simulink library "inp"  (Simulink 8.2)
settings: Solver=ode45  SolverMode=SingleTasking  StartTime=0.0  StopTime=10.0  RelTol=1e-3
undefined symbols (not defined in the file or in its folder): C_TICK_TIME_S, ENUM_FAULTSTATE_FAIL, ENUM_FAULTSTATE_INDETERMINATE, ENUM_FAULTSTATE_PASS, ENUM_KEYSWITCH_ON, FALSE, KINP_ABS1CANCommuOk_flg_ovrdflg, KINP_ABS1CANCommuOk_flg_ovrdval, KINP_ABS1CANPeriodTime_s, KINP_ABS1CANRolCntOk_flg_ovrdflg, KINP_ABS1CANRolCntOk_flg_ovrdval, KINP_ABS2CANChkSumOk_flg_ovrdflg, KINP_ABS2CANChkSumOk_flg_ovrdval, KINP_ABS2CANCommuOk_flg_ovrdflg, KINP_ABS2CANCommuOk_flg_ovrdval, KINP_ABS2CANPeriodTime_s, KINP_ABS2CANRolCntOk_flg_ovrdflg, KINP_ABS2CANRolCntOk_flg_ovrdval, KINP_ABS3CANCommuOk_flg_ovrdflg, KINP_ABS3CANCommuOk_flg_ovrdval, KINP_ABS4CANCommuOk_flg_ovrdflg, KINP_ABS4CANCommuOk_flg_ovrdval, KINP_ABSActive_flg_defval, KINP_ABSActive_flg_invalid, KINP_ABSActive_flg_ovrdflg, KINP_ABSActive_flg_ovrdval, KINP_ABSActive_flg_setvalid, KINP_ABSActive_flg_srvcflg, KINP_ABSActive_flg_srvcval, KINP_ABSCANAlive_flg_ovrdflg, KINP_ABSCANAlive_flg_ovrdval, KINP_ABSCANCommuOk_flg_ovrdflg, KINP_ABSCANCommuOk_flg_ovrdval, KINP_ABSCANValidOk_flg_ovrdflg, KINP_ABSCANValidOk_flg_ovrdval, KINP_ABSFault_flg_defval, KINP_ABSFault_flg_invalid, KINP_ABSFault_flg_ovrdflg, KINP_ABSFault_flg_ovrdval, KINP_ABSFault_flg_setvalid, KINP_ABSFault_flg_srvcflg, KINP_ABSFault_flg_srvcval, KINP_AC1CANCommuOk_flg_ovrdflg, KINP_AC1CANCommuOk_flg_ovrdval, KINP_ACCANAlive_flg_ovrdflg, KINP_ACCANAlive_flg_ovrdval, KINP_ACCANCommuOk_flg_ovrdflg, KINP_ACCANCommuOk_flg_ovrdval, KINP_ACCANValidOk_flg_ovrdflg, KINP_ACCANValidOk_flg_ovrdval, KINP_ACCCancelH_flg_defval, KINP_ACCCancelH_flg_invalid, KINP_ACCCancelH_flg_ovrdflg, KINP_ACCCancelH_flg_ovrdval, KINP_ACCCancelH_flg_setvalid, KINP_ACCCancelH_flg_srvcflg, KINP_ACCCancelH_flg_srvcval, KINP_AccelPedalPosn_pct_defval, KINP_AccelPedalPosn_pct_gain, KINP_AccelPedalPosn_pct_invalid ... (+1866)
libraries: inp x192, hcu_lib x34, simulink x33
(part 14 of 21)

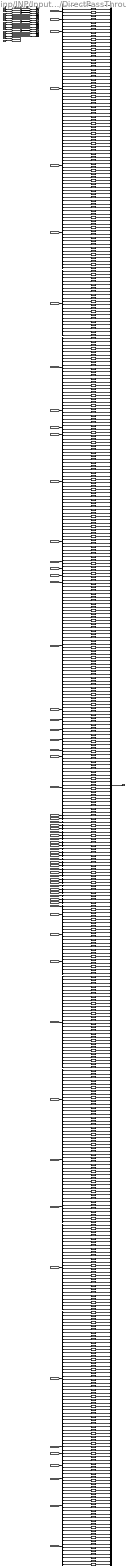
== system inp/INP/InputSignals/DirectPassThrough ==
  ECM_signal_bus : Inport
  TCM_signal_bus : Inport  Port=2
  BCM_signal_bus : Inport  Port=3
  ABS_signal_bus : Inport  Port=4
  EPS_signal_bus : Inport  Port=5
  METER_signal_bus : Inport  Port=6
  AC_signal_bus : Inport  Port=7
  EPB_signal_bus : Inport  Port=8
  CSW_signal_bus : Inport  Port=9
  VCU_signal_bus : Inport  Port=10
  HMI_signal_bus : Inport  Port=11
  EPM_signal_bus : Inport  Port=12
  HWIO_signal_bus : Inport  Port=13
  LLSW_signal_bus : Inport  Port=14
  INP_calc_bus : Inport  Port=15
  INP_diag_bus : Inport  Port=16
  Bus : BusCreator  Inputs=465  OutDataTypeStr=Bus: BINP_outputs
  Bus Selector1 : BusSelector  OutputSignals=VINP_LaneC0LKalF_m_InProc,VINP_LaneC1LKalF_rad_InProc,VINP_LaneC2LKalF_rat_InProc,VINP_LaneC3LKalF_rat_InProc
  Bus Selector10 : BusSelector  OutputSignals=VINP_AckCurBrakDecReq_mps2_InHAL,VINP_AckCurBrakDecReqValid_flg_InHAL,VINP_AckCurBrakDecReqApplable_flg_InHAL,VINP_AckCurBrakDecReqApplableValid_flg_InHAL,VINP_AckCurPTTqReq_Nm_InHAL,VINP_AckCurPTTqReqValid_flg_InHAL,VINP_AckCurPTTqReqApplable_flg_InHAL,VINP_AckCurPTTqReqApplableValid_flg_InHAL,VINP_AckCurVehDec_mps2_InHAL,VINP_AckCurVehDecValid_flg_InHAL,VINP_DeceContManag_flg_InHAL,VINP_DeceContManagValid_flg_InHAL,VINP_DriverBrakPedalOn_flg_InHAL,VINP_DriverBrakPedalOnValid_flg_InHAL,VINP_ECMExtnTqAvailable_flg_InHAL,VINP_ECMExtnTqAvailableValid_flg_InHAL,VINP_ECMTqSource_enum_InHAL,VINP_ECMTqSourceValid_flg_InHAL,VINP_ESCBrakeStatus_enum_InHAL,VINP_ESCBrakeStatusValid_flg_InHAL,VINP_ESCExtnBrkAvailable_flg_InHAL,VINP_ESCExtnBrkAvailableValid_flg_InHAL,VINP_ESCBattVolt_V_InHAL,VINP_ESCBattVoltValid_flg_InHAL,VINP_HWAccelPedalPosn_pct_InHAL,VINP_HWAccelPedalPosnValid_flg_InHAL,VINP_CurDriverTqReq_Nm_InHAL,VINP_CurDriverTqReqValid_flg_InHAL,VINP_TCMGearPosnReqAllow_enum_InHAL,VINP_TCMGearPosnReqAllowValid_flg_InHAL,VINP_MSAStatus_enum_InHAL,VINP_MSAStatusValid_flg_InHAL,VINP_HybridLimpHome_flg_InHAL,VINP_HybridLimpHomeValid_flg_InHAL,VINP_HCUMILReq_flg_InHAL,VINP_HCUMILReqValid_flg_InHAL
  Bus Selector11 : BusSelector  OutputSignals=VINP_ACCTimeGapIncVCU_flg_InHAL,VINP_ACCTimeGapIncVCUValid_flg_InHAL,VINP_ACCTimeGapDecVCU_flg_InHAL,VINP_ACCTimeGapDecVCUValid_flg_InHAL
  Bus Selector12 : BusSelector  OutputSignals=VINP_AEBEnableH_flg_InHAL,VINP_AEBEnableHValid_flg_InHAL,VINP_FCWEnableH_flg_InHAL,VINP_FCWEnableHValid_flg_InHAL,VINP_CSCEnableH_flg_InHAL,VINP_CSCEnableHValid_flg_InHAL,VINP_LKAEnableH_flg_InHAL,VINP_LKAEnableHValid_flg_InHAL,VINP_MAPAEnableH_flg_InHAL,VINP_MAPAEnableHValid_flg_InHAL,VINP_LCSEnableH_flg_InHAL,VINP_LCSEnableHValid_flg_InHAL,VINP_FCWWarningModeH_enum_InHAL,VINP_FCWWarningModeHValid_flg_InHAL,VINP_DefMaxACCSetSpeed_kph_InHAL,VINP_DefMaxACCSetSpeedValid_flg_InHAL
  Bus Selector13 : BusSelector  OutputSignals=VINP_LFCPilotOnHValid_flg_InHAL
  Bus Selector14 : BusSelector  OutputSignals=VINP_LFCPilotOnH_flg_InProc
  Bus Selector15 : BusSelector  OutputSignals=VINP_LFCPilotCancelHValid_flg_InHAL
  Bus Selector16 : BusSelector  OutputSignals=VINP_LFCPilotCancelH_flg_InProc
  Bus Selector17 : BusSelector  OutputSignals=VINP_ACCOnHValid_flg_InHAL
  Bus Selector18 : BusSelector  OutputSignals=VINP_ACCOnH_flg_InProc
  Bus Selector19 : BusSelector  OutputSignals=VINP_ACCCancelHValid_flg_InHAL
  Bus Selector197 : BusSelector  OutputSignals=VINP_IgnitionVolt_V_InHAL,VINP_IgnitionVoltValid_flg_InHAL,VINP_BatteryVolt_V_InHAL,VINP_BatteryVoltValid_flg_InHAL
  Bus Selector2 : BusSelector  OutputSignals=VINP_LaneC0RKalF_m_InProc,VINP_LaneC1RKalF_rad_InProc,VINP_LaneC2RKalF_rat_InProc,VINP_LaneC3RKalF_rat_InProc
  Bus Selector20 : BusSelector  OutputSignals=VINP_ACCCancelH_flg_InProc
  Bus Selector202 : BusSelector  OutputSignals=VINP_IgnitionActive_flg_InProc
  Bus Selector203 : BusSelector  OutputSignals=VHAL_ADCFault_enum,VHAL_EEPROMFault_enum,VHAL_RAMFault_enum,VHAL_ROMFault_enum,VHAL_VREF1Fault_enum,VHAL_VREF2Fault_enum
  Bus Selector204 : BusSelector  OutputSignals=VINP_AccelPedalPosn_pct_InHAL,VINP_AccelPedalPosnValid_flg_InHAL,VINP_EffAccelPedalPosn_pct_InHAL,VINP_EffAccelPedalPosnValid_flg_InHAL,VINP_EngSpd_erpm_InHAL,VINP_EngSpdValid_flg_InHAL,VINP_EngTqFriction_Nm_InHAL,VINP_EngTqFrictionValid_flg_InHAL,VINP_EngTqIndicated_Nm_InHAL,VINP_EngTqIndicatedValid_flg_InHAL,VINP_EngTqIndicatedCOR_Nm_InHAL,VINP_EngTqIndicatedCORValid_flg_InHAL,VINP_EngTqMax_Nm_InHAL,VINP_EngTqMaxValid_flg_InHAL,VINP_EngTqMin_Nm_InHAL,VINP_EngTqMinValid_flg_InHAL,VINP_EngTqUnCORFault_flg_InHAL,VINP_EngTqUnCORFaultValid_flg_InHAL,VINP_EMSHybridOpState_enum_InHAL,VINP_EMSHybridOpStateValid_flg_InHAL,VINP_EMSOpState_enum_InHAL,VINP_EMSOpStateValid_flg_InHAL,VINP_EngHoodOpen_flg_InHAL,VINP_EngHoodOpenValid_flg_InHAL,VINP_OBDWarnCycAchiv_flg_InHAL,VINP_OBDWarnCycAchivValid_flg_InHAL,VINP_OBDDriveCycAchiv_flg_InHAL,VINP_OBDDriveCycAchivValid_flg_InHAL
  Bus Selector209 : BusSelector  OutputSignals=VINP_ABSActive_flg_InHAL,VINP_ABSActiveValid_flg_InHAL,VINP_ABSFault_flg_InHAL,VINP_ABSFaultValid_flg_InHAL,VINP_EBDFault_flg_InHAL,VINP_EBDFaultValid_flg_InHAL,VINP_ESCActive_flg_InHAL,VINP_ESCActiveValid_flg_InHAL,VINP_ESCFault_flg_InHAL,VINP_ESCFaultValid_flg_InHAL,VINP_TCSActive_flg_InHAL,VINP_TCSActiveValid_flg_InHAL,VINP_TCSEnabled_flg_InHAL,VINP_TCSEnabledValid_flg_InHAL,VINP_TCSFault_flg_InHAL,VINP_TCSFaultValid_flg_InHAL,VINP_HSAActive_flg_InHAL,VINP_HSAActiveValid_flg_InHAL,VINP_HSAFault_flg_InHAL,VINP_HSAFaultValid_flg_InHAL
  Bus Selector21 : BusSelector  OutputSignals=VINP_ACCResSpdIncHValid_flg_InHAL
  Bus Selector22 : BusSelector  OutputSignals=VINP_ACCResSpdIncH_flg_InProc
  Bus Selector220 : BusSelector  OutputSignals=VINP_VehSpd_mps_InProc,VINP_SteerAngle_rad_InProc,VINP_YawRate_radps_InProc
  Bus Selector226 : BusSelector  OutputSignals=VINP_KeySwitchStatus_enum_InHAL,VINP_KeySwitchStatusValid_flg_InHAL,VINP_TurnSignalSwL_flg_InHAL,VINP_TurnSignalSwLValid_flg_InHAL,VINP_TurnSignalSwR_flg_InHAL,VINP_TurnSignalSwRValid_flg_InHAL,VINP_HazardLightSw_flg_InHAL,VINP_HazardLightSwValid_flg_InHAL,VINP_DriverDoorOpen_flg_InHAL,VINP_DriverDoorOpenValid_flg_InHAL,VINP_PassengerDoorOpen_flg_InHAL,VINP_PassengerDoorOpenValid_flg_InHAL,VINP_LRDoorOpen_flg_InHAL,VINP_LRDoorOpenValid_flg_InHAL,VINP_RRDoorOpen_flg_InHAL,VINP_RRDoorOpenValid_flg_InHAL,VINP_TailGateOpen_flg_InHAL,VINP_TailGateOpenValid_flg_InHAL,VINP_AnyDoorOpen_flg_InHAL,VINP_AnyDoorOpenValid_flg_InHAL,VINP_VehCrashed_flg_InHAL,VINP_VehCrashedValid_flg_InHAL
  Bus Selector23 : BusSelector  OutputSignals=VINP_ACCSetSpdDecHValid_flg_InHAL
  Bus Selector230 : BusSelector  OutputSignals=VINP_ShifterPosn_enum_InHAL,VINP_ShifterPosnValid_flg_InHAL,VINP_NowGearPosn_enum_InHAL,VINP_NowGearPosnValid_flg_InHAL,VINP_TMTurbineSpd_rpm_InHAL,VINP_TMTurbineSpdValid_flg_InHAL,VINP_TCMFaultStatus_enum_InHAL,VINP_TCMFaultStatusValid_flg_InHAL,VINP_TqConvClutchSt_enum_InHAL,VINP_TqConvClutchStValid_flg_InHAL,VINP_TCMMILReq_flg_InHAL,VINP_TCMMILReqValid_flg_InHAL,VINP_TCMWinterMode_flg_InHAL,VINP_TCMWinterModeValid_flg_InHAL,VINP_TCMPowerMode_flg_InHAL,VINP_TCMPowerModeValid_flg_InHAL,VINP_DriverMode_enum_InHAL,VINP_DriverModeValid_flg_InHAL
  Bus Selector232 : BusSelector  OutputSignals=VINP_RelSteerAngle_deg_InHAL,VINP_RelSteerAngleValid_flg_InHAL,VINP_SteerTorque_Nm_InHAL,VINP_SteerTorqueValid_flg_InHAL,VINP_EPSElectPWR_A_InHAL,VINP_EPSElectPWRValid_flg_InHAL,VINP_EPSFailed_flg_InHAL,VINP_EPSFailedValid_flg_InHAL,VINP_AckCurStrAngReq_deg_InHAL,VINP_AckCurStrAngReqValid_flg_InHAL,VINP_AckCurStrAngReqApplable_flg_InHAL,VINP_AckCurStrAngReqApplableValid_flg_InHAL,VINP_AckCurStrTqReq_Nm_InHAL,VINP_AckCurStrTqReqValid_flg_InHAL,VINP_AckCurStrTqReqApplable_flg_InHAL,VINP_AckCurStrTqReqApplableValid_flg_InHAL,VINP_EPSBattVolt_V_InHAL,VINP_EPSBattVoltValid_flg_InHAL,VINP_EPASMILReq_flg_InHAL,VINP_EPASMILReqValid_flg_InHAL,VINP_EPSLKAStatus_enum_InHAL,VINP_EPSLKAStatusValid_flg_InHAL,VINP_EPSLFCStatus_enum_InHAL,VINP_EPSLFCStatusValid_flg_InHAL,VINP_EPSADASAbReason_enum_InHAL,VINP_EPSADASAbReasonValid_flg_InHAL
  Bus Selector237 : BusSelector  OutputSignals=VINP_LaneQualityL_enum_InHAL,VINP_LaneQualityLValid_flg_InHAL,VINP_LaneQualityR_enum_InHAL,VINP_LaneQualityRValid_flg_InHAL
  Bus Selector239 : BusSelector  OutputSignals=VINP_LaneC0L_m_InHAL,VINP_LaneC0LValid_flg_InHAL,VINP_LaneC1L_rad_InHAL,VINP_LaneC1LValid_flg_InHAL,VINP_LaneC2L_rat_InHAL,VINP_LaneC2LValid_flg_InHAL,VINP_LaneC3L_rat_InHAL,VINP_LaneC3LValid_flg_InHAL
  Bus Selector24 : BusSelector  OutputSignals=VINP_ACCSetSpdDecH_flg_InProc
  Bus Selector240 : BusSelector  OutputSignals=VINP_LaneC0R_m_InHAL,VINP_LaneC0RValid_flg_InHAL,VINP_LaneC1R_rad_InHAL,VINP_LaneC1RValid_flg_InHAL,VINP_LaneC2R_rat_InHAL,VINP_LaneC2RValid_flg_InHAL,VINP_LaneC3R_rat_InHAL,VINP_LaneC3RValid_flg_InHAL
  Bus Selector241 : BusSelector  OutputSignals=VINP_ABSCANValid_flg_InProc,VINP_BCMCANValid_flg_InProc,VINP_ECMCANValid_flg_InProc,VINP_TCMCANValid_flg_InProc,VINP_EPSCANValid_flg_InProc,VINP_METERCANValid_flg_InProc,VINP_ACCANValid_flg_InProc,VINP_EPBCANValid_flg_InProc,VINP_VCUCANValid_flg_InProc,VINP_HMICANValid_flg_InProc,VINP_CSWCANValid_flg_InProc,VINP_EPMCANValid_flg_InProc
  Bus Selector242 : BusSelector  OutputSignals=VINP_ABSCANAlive_flg_InProc,VINP_BCMCANAlive_flg_InProc,VINP_ECMCANAlive_flg_InProc,VINP_TCMCANAlive_flg_InProc,VINP_EPSCANAlive_flg_InProc,VINP_METERCANAlive_flg_InProc,VINP_ACCANAlive_flg_InProc,VINP_EPBCANAlive_flg_InProc,VINP_VCUCANAlive_flg_InProc,VINP_HMICANAlive_flg_InProc,VINP_CSWCANAlive_flg_InProc,VINP_EPMCANAlive_flg_InProc
  Bus Selector25 : BusSelector  OutputSignals=VINP_ACCSpdIncHValid_flg_InHAL
  Bus Selector26 : BusSelector  OutputSignals=VINP_ACCSpdIncH_flg_InProc
  Bus Selector27 : BusSelector  OutputSignals=VINP_ACCSpdDecHValid_flg_InHAL
  Bus Selector28 : BusSelector  OutputSignals=VINP_ACCSpdDecH_flg_InProc
  Bus Selector29 : BusSelector  OutputSignals=VINP_ACCTimeGapIncHValid_flg_InHAL
  Bus Selector3 : BusSelector  OutputSignals=VINP_LFWheelSpeed_kph_InHAL,VINP_LFWheelSpeedValid_flg_InHAL,VINP_LRWheelSpeed_kph_InHAL,VINP_LRWheelSpeedValid_flg_InHAL,VINP_RFWheelSpeed_kph_InHAL,VINP_RFWheelSpeedValid_flg_InHAL,VINP_RRWheelSpeed_kph_InHAL,VINP_RRWheelSpeedValid_flg_InHAL
  Bus Selector30 : BusSelector  OutputSignals=VINP_ACCTimeGapIncH_flg_InProc
  Bus Selector31 : BusSelector  OutputSignals=VINP_ACCTimeGapDecHValid_flg_InHAL
  Bus Selector32 : BusSelector  OutputSignals=VINP_ACCTimeGapDecH_flg_InProc
  Bus Selector33 : BusSelector  OutputSignals=VINP_IGSAOnHValid_flg_InHAL
  Bus Selector34 : BusSelector  OutputSignals=VINP_IGSAOnH_flg_InProc
  Bus Selector35 : BusSelector  OutputSignals=VINP_IGSAResSpdIncHValid_flg_InHAL
  Bus Selector36 : BusSelector  OutputSignals=VINP_IGSAResSpdIncH_flg_InProc
  Bus Selector37 : BusSelector  OutputSignals=VINP_IGSASetSpdDecHValid_flg_InHAL
  Bus Selector38 : BusSelector  OutputSignals=VINP_IGSASetSpdDecH_flg_InProc
  Bus Selector39 : BusSelector  OutputSignals=VINP_IGSASwitchModeHValid_flg_InHAL
  Bus Selector4 : BusSelector  OutputSignals=VINP_AppTime_ms_InProc
  Bus Selector40 : BusSelector  OutputSignals=VINP_IGSASwitchModeH_flg_InProc
  Bus Selector41 : BusSelector  OutputSignals=VINP_SteerAngle_deg_InHAL,VINP_SteerAngleValid_flg_InHAL
  Bus Selector42 : BusSelector  OutputSignals=VINP_SteerSpeed_degps_InProc,VINP_SteerSpeedValid_flg_InProc
  Bus Selector43 : BusSelector  OutputSignals=VINP_HighBeamSwOn_flg_InHAL,VINP_HighBeamSwOnValid_flg_InHAL
  Bus Selector44 : BusSelector  OutputSignals=VINP_ACCResSpdIncVCU_flg_InHAL,VINP_ACCResSpdIncVCUValid_flg_InHAL,VINP_ACCSetSpdDecVCU_flg_InHAL,VINP_ACCSetSpdDecVCUValid_flg_InHAL
  Bus Selector45 : BusSelector  OutputSignals=VINP_ACCOnVCU_flg_InProc,VINP_ACCOnVCUValid_flg_InProc
  Bus Selector46 : BusSelector  OutputSignals=VINP_ACCDistVCU_flg_InProc,VINP_ACCDistVCUValid_flg_InProc
  Bus Selector47 : BusSelector  OutputSignals=VINP_CamNofObjects_num_InHAL,VINP_CamNofObjectsValid_flg_InHAL,VINP_CamGoDecision_enum_InHAL,VINP_CamGoDecisionValid_flg_InHAL,VINP_CloseCarDetected_flg_InHAL,VINP_CloseCarDetectedValid_flg_InHAL,VINP_RightCarCutIn_flg_InHAL,VINP_RightCarCutInValid_flg_InHAL,VINP_LeftCarCutIn_flg_InHAL,VINP_LeftCarCutInValid_flg_InHAL
  Bus Selector48 : BusSelector  OutputSignals=VINP_CamFSImpairedVD_flg_InHAL,VINP_CamFSImpairedVDValid_flg_InHAL,VINP_CamFSLowSun_flg_InHAL,VINP_CamFSLowSunValid_flg_InHAL,VINP_CamFSBlurImage_flg_InHAL,VINP_CamFSBlurImageValid_flg_InHAL,VINP_CamFSSmearImage_flg_InHAL,VINP_CamFSSmearImageValid_flg_InHAL,VINP_CamFSPartialBlockage_flg_InHAL,VINP_CamFSPartialBlockageValid_flg_InHAL,VINP_CamFSFullBlockage_flg_InHAL,VINP_CamFSFullBlockageValid_flg_InHAL,VINP_CamFSSplashes_flg_InHAL,VINP_CamFSSplashesValid_flg_InHAL,VINP_CamFSOutofCal_flg_InHAL,VINP_CamFSOutofCalValid_flg_InHAL,VINP_CamFSCalMisalign_flg_InHAL,VINP_CamFSCalMisalignValid_flg_InHAL,VINP_CamFSSmearedspots_flg_InHAL,VINP_CamFSSmearedspotsValid_flg_InHAL,VINP_CamFSSpotHalosFrozen_flg_InHAL,VINP_CamFSSpotHalosFrozenValid_flg_InHAL,VINP_CamFSFoggySpots_flg_InHAL,VINP_CamFSFoggySpotsValid_flg_InHAL,VINP_CamFSSunRay_flg_InHAL,VINP_CamFSSunRayValid_flg_InHAL
  Bus Selector49 : BusSelector  OutputSignals=VINP_RearFogSwOn_flg_InProc,VINP_RearFogSwOnValid_flg_InProc
  Bus Selector5 : BusSelector  OutputSignals=VINP_LaneTypeL_enum_InHAL,VINP_LaneTypeLValid_flg_InHAL,VINP_LaneTypeR_enum_InHAL,VINP_LaneTypeRValid_flg_InHAL,VINP_LaneMrkClrL_enum_InHAL,VINP_LaneMrkClrLValid_flg_InHAL,VINP_LaneMrkClrR_enum_InHAL,VINP_LaneMrkClrRValid_flg_InHAL,VINP_LaneMrkWidL_m_InHAL,VINP_LaneMrkWidLValid_flg_InHAL,VINP_LaneMrkWidR_m_InHAL,VINP_LaneMrkWidRValid_flg_InHAL,VINP_ViewRangeStartL_m_InHAL,VINP_ViewRangeStartLValid_flg_InHAL,VINP_ViewRangeStartR_m_InHAL,VINP_ViewRangeStartRValid_flg_InHAL,VINP_ViewRangeEndL_m_InHAL,VINP_ViewRangeEndLValid_flg_InHAL,VINP_ViewRangeEndR_m_InHAL,VINP_ViewRangeEndRValid_flg_InHAL,VINP_TLCL_s_InHAL,VINP_TLCLValid_flg_InHAL,VINP_TLCR_s_InHAL,VINP_TLCRValid_flg_InHAL,VINP_LaneCrosL_flg_InHAL,VINP_LaneCrosLValid_flg_InHAL,VINP_LaneCrosR_flg_InHAL,VINP_LaneCrosRValid_flg_InHAL
  Bus Selector50 : BusSelector  OutputSignals=VINP_LFCOnVCU_flg_InProc,VINP_LFCOnVCUValid_flg_InProc
  Bus Selector51 : BusSelector  OutputSignals=VINP_MSAOnVCU_flg_InHAL,VINP_MSAOnVCUValid_flg_InHAL
  Bus Selector52 : BusSelector  OutputSignals=VINP_CamObsID10_num_InProc,VINP_CamObsIDValid10_flg_InProc,VINP_CamObsPosX10_m_InProc,VINP_CamObsPosXValid10_flg_InProc,VINP_CamObsPosY10_m_InProc,VINP_CamObsPosYValid10_flg_InProc,VINP_CamObsVelX10_mps_InProc,VINP_CamObsVelXValid10_flg_InProc,VINP_CamObsVelY10_mps_InProc,VINP_CamObsVelYValid10_flg_InProc,VINP_CamObsAccX10_mps2_InProc,VINP_CamObsAccXValid10_flg_InProc,VINP_CamObsAng10_deg_InProc,VINP_CamObsAngValid10_flg_InProc,VINP_CamObsAngRate10_degps_InProc,VINP_CamObsAngRateValid10_flg_InProc,VINP_CamObsLength10_m_InProc,VINP_CamObsLengthValid10_flg_InProc,VINP_CamObsWidth10_m_InProc,VINP_CamObsWidthValid10_flg_InProc,VINP_CamObsType10_enum_InProc,VINP_CamObsTypeValid10_flg_InProc,VINP_CamObsStatus10_enum_InProc,VINP_CamObsStatusValid10_flg_InProc,VINP_CamObsValid10_enum_InProc,VINP_CamObsValidValid10_flg_InProc,VINP_CamObsMoveInO10_enum_InProc,VINP_CamObsMoveInOValid10_flg_InProc,VINP_CamObsBlinker10_enum_InProc,VINP_CamObsBlinkerValid10_flg_InProc,VINP_CamObsBrakeLight10_flg_InProc,VINP_CamObsBrakeLightValid10_flg_InProc,VINP_CamObsAge10_cnt_InProc,VINP_CamObsAgeValid10_flg_InProc,VINP_CamObsLane10_enum_InProc,VINP_CamObsLaneValid10_flg_InProc,VINP_CamObsCIPV10_flg_InProc,VINP_CamObsCIPVValid10_flg_InProc,VINP_CamObsReplaced10_flg_InProc,VINP_CamObsReplacedValid10_flg_InProc
  Bus Selector53 : BusSelector  OutputSignals=VINP_LeftNextLane_BUS_InHAL.VINP_NextLaneC0L_m_InHAL,VINP_LeftNextLane_BUS_InHAL.VINP_NextLaneC0LValid_flg_InHAL,VINP_LeftNextLane_BUS_InHAL.VINP_NextLaneC1L_rad_InHAL,VINP_LeftNextLane_BUS_InHAL.VINP_NextLaneC1LValid_flg_InHAL,VINP_LeftNextLane_BUS_InHAL.VINP_NextLaneC2L_rat_InHAL,VINP_LeftNextLane_BUS_InHAL.VINP_NextLaneC2LValid_flg_InHAL,VINP_LeftNextLane_BUS_InHAL.VINP_NextLaneC3L_rat_InHAL,VINP_LeftNextLane_BUS_InHAL.VINP_NextLaneC3LValid_flg_InHAL,VINP_LeftNextLane_BUS_InHAL.VINP_NextLaneQualityL_enum_InHAL,VINP_LeftNextLane_BUS_InHAL.VINP_NextLaneQualityLValid_flg_InHAL,VINP_LeftNextLane_BUS_InHAL.VINP_NextLaneTypeL_enum_InHAL,VINP_LeftNextLane_BUS_InHAL.VINP_NextLaneTypeLValid_flg_InHAL,VINP_LeftNextLane_BUS_InHAL.VINP_NextLaneMrkWidL_m_InHAL,VINP_LeftNextLane_BUS_InHAL.VINP_NextLaneMrkWidLValid_flg_InHAL,VINP_LeftNextLane_BUS_InHAL.VINP_NextViewRangeStartL_m_InHAL,VINP_LeftNextLane_BUS_InHAL.VINP_NextViewRangeStartLValid_flg_InHAL,VINP_LeftNextLane_BUS_InHAL.VINP_NextViewRangeEndL_m_InHAL,VINP_LeftNextLane_BUS_InHAL.VINP_NextViewRangeEndLValid_flg_InHAL
  Bus Selector54 : BusSelector  OutputSignals=VINP_RightNextLane_BUS_InHAL.VINP_NextLaneC0R_m_InHAL,VINP_RightNextLane_BUS_InHAL.VINP_NextLaneC0RValid_flg_InHAL,VINP_RightNextLane_BUS_InHAL.VINP_NextLaneC1R_rad_InHAL,VINP_RightNextLane_BUS_InHAL.VINP_NextLaneC1RValid_flg_InHAL,VINP_RightNextLane_BUS_InHAL.VINP_NextLaneC2R_rat_InHAL,VINP_RightNextLane_BUS_InHAL.VINP_NextLaneC2RValid_flg_InHAL,VINP_RightNextLane_BUS_InHAL.VINP_NextLaneC3R_rat_InHAL,VINP_RightNextLane_BUS_InHAL.VINP_NextLaneC3RValid_flg_InHAL,VINP_RightNextLane_BUS_InHAL.VINP_NextLaneQualityR_enum_InHAL,VINP_RightNextLane_BUS_InHAL.VINP_NextLaneQualityRValid_flg_InHAL,VINP_RightNextLane_BUS_InHAL.VINP_NextLaneTypeR_enum_InHAL,VINP_RightNextLane_BUS_InHAL.VINP_NextLaneTypeRValid_flg_InHAL,VINP_RightNextLane_BUS_InHAL.VINP_NextLaneMrkWidR_m_InHAL,VINP_RightNextLane_BUS_InHAL.VINP_NextLaneMrkWidRValid_flg_InHAL,VINP_RightNextLane_BUS_InHAL.VINP_NextViewRangeStartR_m_InHAL,VINP_RightNextLane_BUS_InHAL.VINP_NextViewRangeStartRValid_flg_InHAL,VINP_RightNextLane_BUS_InHAL.VINP_NextViewRangeEndR_m_InHAL,VINP_RightNextLane_BUS_InHAL.VINP_NextViewRangeEndRValid_flg_InHAL
  Bus Selector6 : BusSelector  OutputSignals=VINP_OdoMeter_km_InHAL,VINP_OdoMeterValid_flg_InHAL,VINP_LowBrakeFluid_flg_InHAL,VINP_LowBrakeFluidValid_flg_InHAL,VINP_ParkBrakeOn_flg_InHAL,VINP_ParkBrakeOnValid_flg_InHAL,VINP_SeatBeltNOTFasten_flg_InHAL,VINP_SeatBeltNOTFastenValid_flg_InHAL,VINP_MeterVehSpd_kph_InHAL,VINP_MeterVehSpdValid_flg_InHAL
  Bus Selector7 : BusSelector  OutputSignals=VINP_BrkLampOn_flg_InHAL,VINP_BrkLampOnValid_flg_InHAL,VINP_VehSpd_kph_InHAL,VINP_VehSpdValid_flg_InHAL,VINP_YawRate_degps_InHAL,VINP_YawRateValid_flg_InHAL,VINP_LatAccel_G_InHAL,VINP_LatAccelValid_flg_InHAL,VINP_LongAccel_G_InHAL,VINP_LongAccelValid_flg_InHAL,VINP_MCPressure_bar_InHAL,VINP_MCPressureValid_flg_InHAL,VINP_SurfaceGrade_deg_InHAL,VINP_SurfaceGradeValid_flg_InHAL,VINP_BrakBoosterPres_kPa_InHAL,VINP_BrakBoosterPresValid_flg_InHAL,VINP_LowBrakBoosterPres_flg_InHAL,VINP_LowBrakBoosterPresValid_flg_InHAL
  Bus Selector8 : BusSelector  OutputSignals=VINP_OutsideTemp_degC_InHAL,VINP_OutsideTempValid_flg_InHAL
  Bus Selector9 : BusSelector  OutputSignals=VINP_ParkBrakeBindSta_enum_InHAL,VINP_ParkBrakeBindStaValid_flg_InHAL
  Cast{1,3..6,13..23,25,27..28,32,34,36,38,40,42,45,56..69,78..88,90..92,94..105,108,110,112,114,116,118,120,122,124,126..130,132,134,136,138,140,142,144..145,147,149,151..153,155..157,159..161,164,166..167,169..175,177,180,182..185,188,190..193,195,197..201,203,205,207,209..221,224..232,234..236,238..240,242,244..250,252,254..256,258,260,262..264,266,268,270..316,318..330,332,334..336,338,340..342,344..389,391,393,395,397,399,401,403,405,407,409,411,413,415,417,419..421,423,425..429,432..433,435,437,439,441,443,445,447,450..451,453,455,457,459,461,463,465} (331 alike) : DataTypeConversion  OutDataTypeStr=boolean  RndMeth=Zero
  Cast{8..12,30,46..47,93,106..107,109,111,113,141,163,165,179,181,194,196,202,206,208,243,251,253,261,269,317,339,390,410,412,414,416,418,422,424,438,440,456,458} (43 alike) : DataTypeConversion  OutDataTypeStr=uint8  RndMeth=Zero
  Cast{2,7,24,26,29,31,33,35,37,39,41,43..44,48..55,70..77,89,115,117,119,121,123,125,131,133,135,137,139,143,146,148,150,154,158,162,168,176,178,186..187,189,204,222..223,233,237,241,257,259,265,267,331,333,337,343,392,394,396,398,400,402,404,406,408,430..431,434,436,442,444,446,448..449,452,454,460,462,464} (91 alike) : DataTypeConversion  OutDataTypeStr=single  RndMeth=Zero
  Terminator{,1..13} (14 alike) : Terminator
  BINP_outputs : Outport  OutDataTypeStr=Bus: BINP_outputs
  wires:
  INP_calc_bus/1 -> [INP_calc_bus]
  Bus/1 -> BINP_outputs/1  "BINP_outputs"
  Cast2/1 -> Bus/1  "VINP_IgnitionVolt_V"
  Cast3/1 -> Bus/2  "VINP_IgnitionVoltValid_flg"
  Cast6/1 -> Bus/430  "VINP_IgnitionActive_flg"
  Cast7/1 -> Bus/5  "VINP_AppTime_ms"
  Cast29/1 -> Bus/431  "VINP_VehSpd_mps"
  Cast31/1 -> Bus/432  "VINP_SteerAngle_rad"
  Cast33/1 -> Bus/433  "VINP_YawRate_radps"
  Cast46/1 -> Bus/270  "VINP_LaneQualityL_enum"
  Cast47/1 -> Bus/272  "VINP_LaneQualityR_enum"
  Cast48/1 -> Bus/274  "VINP_LaneC0LRaw_m"
  Cast49/1 -> Bus/276  "VINP_LaneC1LRaw_rad"
  Cast50/1 -> Bus/278  "VINP_LaneC2LRaw_rat"
  Cast51/1 -> Bus/280  "VINP_LaneC3LRaw_rat"
  Cast52/1 -> Bus/282  "VINP_LaneC0RRaw_m"
  Cast53/1 -> Bus/284  "VINP_LaneC1RRaw_rad"
  Cast54/1 -> Bus/286  "VINP_LaneC2RRaw_rat"
  Cast55/1 -> Bus/288  "VINP_LaneC3RRaw_rat"
  LLSW_signal_bus/1 -> [LLSW_signal_bus]
  HWIO_signal_bus/1 -> [HWIO_signal_bus]
  EPM_signal_bus/1 -> [EPM_signal_bus]
  EPS_signal_bus/1 -> [EPS_signal_bus]
  ABS_signal_bus/1 -> [ABS_signal_bus]
  BCM_signal_bus/1 -> [BCM_signal_bus]
  TCM_signal_bus/1 -> [TCM_signal_bus]
  ECM_signal_bus/1 -> [ECM_signal_bus]
  [ECM_signal_bus] -> Terminator/1
  [TCM_signal_bus] -> Terminator1/1
  [BCM_signal_bus] -> Terminator2/1
  [ABS_signal_bus] -> Terminator3/1
  [EPS_signal_bus] -> Terminator4/1
  [EPM_signal_bus] -> Terminator6/1
  [HWIO_signal_bus] -> Terminator7/1
  [LLSW_signal_bus] -> Terminator8/1
  Bus Selector197/1 -> Cast2/1  "<VINP_IgnitionVolt_V_InHAL>"
  Bus Selector197/2 -> Cast3/1  "<VINP_IgnitionVoltValid_flg_InHAL>"
  [INP_calc_bus] -> Bus Selector202/1
  Bus Selector202/1 -> Cast6/1  "<VINP_IgnitionActive_flg_InProc>"
  [LLSW_signal_bus] -> Bus Selector203/1
  Bus Selector4/1 -> Cast7/1  "<VINP_AppTime_ms_InProc>"
  [INP_calc_bus] -> Bus Selector220/1
  Bus Selector220/1 -> Cast29/1  "<VINP_VehSpd_mps_InProc>"
  Bus Selector220/2 -> Cast31/1  "<VINP_SteerAngle_rad_InProc>"
  Bus Selector220/3 -> Cast33/1  "<VINP_YawRate_radps_InProc>"
  [EPM_signal_bus] -> Bus Selector237/1
  Bus Selector237/1 -> Cast46/1  "<VINP_LaneQualityL_enum_InHAL>"
  Bus Selector237/3 -> Cast47/1  "<VINP_LaneQualityR_enum_InHAL>"
  [EPM_signal_bus] -> Bus Selector239/1
  Bus Selector239/1 -> Cast48/1  "<VINP_LaneC0L_m_InHAL>"
  Bus Selector239/3 -> Cast49/1  "<VINP_LaneC1L_rad_InHAL>"
  Bus Selector239/5 -> Cast50/1  "<VINP_LaneC2L_rat_InHAL>"
  Bus Selector239/7 -> Cast51/1  "<VINP_LaneC3L_rat_InHAL>"
  [EPM_signal_bus] -> Bus Selector240/1
  Bus Selector240/1 -> Cast52/1  "<VINP_LaneC0R_m_InHAL>"
  Bus Selector240/3 -> Cast53/1  "<VINP_LaneC1R_rad_InHAL>"
  Bus Selector240/5 -> Cast54/1  "<VINP_LaneC2R_rat_InHAL>"
  Bus Selector240/7 -> Cast55/1  "<VINP_LaneC3R_rat_InHAL>"
  [HWIO_signal_bus] -> Bus Selector197/1
  Cast70/1 -> Bus/434  "VINP_LaneC0LKalF_m"
  Cast71/1 -> Bus/435  "VINP_LaneC1LKalF_rad"
  Cast72/1 -> Bus/436  "VINP_LaneC2LKalF_rat"
  Cast73/1 -> Bus/437  "VINP_LaneC3LKalF_rat"
  Cast74/1 -> Bus/438  "VINP_LaneC0RKalF_m"
  Cast75/1 -> Bus/439  "VINP_LaneC1RKalF_rad"
  Cast76/1 -> Bus/440  "VINP_LaneC2RKalF_rat"
  Cast77/1 -> Bus/441  "VINP_LaneC3RKalF_rat"
  [INP_calc_bus] -> Bus Selector1/1
  Bus Selector1/1 -> Cast70/1  "<VINP_LaneC0LKalF_m_InProc>"
  Bus Selector1/2 -> Cast71/1  "<VINP_LaneC1LKalF_rad_InProc>"
  Bus Selector1/3 -> Cast72/1  "<VINP_LaneC2LKalF_rat_InProc>"
  Bus Selector1/4 -> Cast73/1  "<VINP_LaneC3LKalF_rat_InProc>"
  [INP_calc_bus] -> Bus Selector2/1
  Bus Selector2/1 -> Cast74/1  "<VINP_LaneC0RKalF_m_InProc>"
  Bus Selector2/2 -> Cast75/1  "<VINP_LaneC1RKalF_rad_InProc>"
  Bus Selector2/3 -> Cast76/1  "<VINP_LaneC2RKalF_rat_InProc>"
  Bus Selector2/4 -> Cast77/1  "<VINP_LaneC3RKalF_rat_InProc>"
  INP_diag_bus/1 -> [INP_diag_bus]
  Cast11/1 -> Bus/10  "VINP_VREF1Fault_enum"
  Cast12/1 -> Bus/11  "VINP_VREF2Fault_enum"
  Bus Selector203/5 -> Cast11/1  "<VHAL_VREF1Fault_enum>"
  Bus Selector203/6 -> Cast12/1  "<VHAL_VREF2Fault_enum>"
  Cast8/1 -> Bus/7  "VINP_EEPROMFault_enum"
  Cast9/1 -> Bus/8  "VINP_RAMFault_enum"
  Cast10/1 -> Bus/9  "VINP_ROMFault_enum"
  Bus Selector203/2 -> Cast8/1  "<VHAL_EEPROMFault_enum>"
  Bus Selector203/3 -> Cast9/1  "<VHAL_RAMFault_enum>"
  Bus Selector203/4 -> Cast10/1  "<VHAL_ROMFault_enum>"
  Bus Selector237/2 -> Cast96/1  "<VINP_LaneQualityLValid_flg_InHAL>"
  Cast96/1 -> Bus/271  "VINP_LaneQualityLValid_flg"
  Bus Selector237/4 -> Cast97/1  "<VINP_LaneQualityRValid_flg_InHAL>"
  Cast97/1 -> Bus/273  "VINP_LaneQualityRValid_flg"
  Bus Selector239/2 -> Cast98/1  "<VINP_LaneC0LValid_flg_InHAL>"
  Cast98/1 -> Bus/275  "VINP_LaneC0LValid_flg"
  Bus Selector239/4 -> Cast99/1  "<VINP_LaneC1LValid_flg_InHAL>"
  Cast99/1 -> Bus/277  "VINP_LaneC1LValid_flg"
  Bus Selector239/6 -> Cast100/1  "<VINP_LaneC2LValid_flg_InHAL>"
  Cast100/1 -> Bus/279  "VINP_LaneC2LValid_flg"
  Bus Selector239/8 -> Cast101/1  "<VINP_LaneC3LValid_flg_InHAL>"
  Cast101/1 -> Bus/281  "VINP_LaneC3LValid_flg"
  Bus Selector240/2 -> Cast102/1  "<VINP_LaneC0RValid_flg_InHAL>"
  Cast102/1 -> Bus/283  "VINP_LaneC0RValid_flg"
  Bus Selector240/4 -> Cast103/1  "<VINP_LaneC1RValid_flg_InHAL>"
  Cast103/1 -> Bus/285  "VINP_LaneC1RValid_flg"
  Bus Selector240/6 -> Cast104/1  "<VINP_LaneC2RValid_flg_InHAL>"
  Cast104/1 -> Bus/287  "VINP_LaneC2RValid_flg"
  Bus Selector240/8 -> Cast105/1  "<VINP_LaneC3RValid_flg_InHAL>"
  Cast105/1 -> Bus/289  "VINP_LaneC3RValid_flg"
  Bus Selector203/1 -> Cast106/1  "<VHAL_ADCFault_enum>"
  Cast106/1 -> Bus/6  "VINP_ADCFault_enum"
  [INP_calc_bus] -> Bus Selector4/1
  [EPM_signal_bus] -> Bus Selector5/1
  Bus Selector5/1 -> Cast107/1  "<VINP_LaneTypeL_enum_InHAL>"
  Bus Selector5/2 -> Cast108/1  "<VINP_LaneTypeLValid_flg_InHAL>"
  Bus Selector5/3 -> Cast109/1  "<VINP_LaneTypeR_enum_InHAL>"
  Bus Selector5/4 -> Cast110/1  "<VINP_LaneTypeRValid_flg_InHAL>"
  Bus Selector5/5 -> Cast111/1  "<VINP_LaneMrkClrL_enum_InHAL>"
  Bus Selector5/6 -> Cast112/1  "<VINP_LaneMrkClrLValid_flg_InHAL>"
  Bus Selector5/7 -> Cast113/1  "<VINP_LaneMrkClrR_enum_InHAL>"
  Bus Selector5/8 -> Cast114/1  "<VINP_LaneMrkClrRValid_flg_InHAL>"
  Bus Selector5/9 -> Cast115/1  "<VINP_LaneMrkWidL_m_InHAL>"
  Bus Selector5/10 -> Cast116/1  "<VINP_LaneMrkWidLValid_flg_InHAL>"
  Bus Selector5/11 -> Cast117/1  "<VINP_LaneMrkWidR_m_InHAL>"
  Bus Selector5/12 -> Cast118/1  "<VINP_LaneMrkWidRValid_flg_InHAL>"
  Bus Selector5/13 -> Cast119/1  "<VINP_ViewRangeStartL_m_InHAL>"
  Bus Selector5/14 -> Cast120/1  "<VINP_ViewRangeStartLValid_flg_InHAL>"
  Bus Selector5/15 -> Cast121/1  "<VINP_ViewRangeStartR_m_InHAL>"
  Bus Selector5/16 -> Cast122/1  "<VINP_ViewRangeStartRValid_flg_InHAL>"
  Bus Selector5/21 -> Cast123/1  "<VINP_TLCL_s_InHAL>"
  Bus Selector5/22 -> Cast124/1  "<VINP_TLCLValid_flg_InHAL>"
  Bus Selector5/23 -> Cast125/1  "<VINP_TLCR_s_InHAL>"
  Bus Selector5/24 -> Cast126/1  "<VINP_TLCRValid_flg_InHAL>"
  Bus Selector5/25 -> Cast127/1  "<VINP_LaneCrosL_flg_InHAL>"
  Bus Selector5/26 -> Cast128/1  "<VINP_LaneCrosLValid_flg_InHAL>"
  Bus Selector5/27 -> Cast129/1  "<VINP_LaneCrosR_flg_InHAL>"
  Bus Selector5/28 -> Cast130/1  "<VINP_LaneCrosRValid_flg_InHAL>"
  Cast107/1 -> Bus/290  "VINP_LaneTypeL_enum"
  Cast108/1 -> Bus/291  "VINP_LaneTypeLValid_flg"
  Cast109/1 -> Bus/292  "VINP_LaneTypeR_enum"
  Cast110/1 -> Bus/293  "VINP_LaneTypeRValid_flg"
  Cast111/1 -> Bus/294  "VINP_LaneMrkClrL_enum"
  Cast112/1 -> Bus/295  "VINP_LaneMrkClrLValid_flg"
  Cast113/1 -> Bus/296  "VINP_LaneMrkClrR_enum"
  Cast114/1 -> Bus/297  "VINP_LaneMrkClrRValid_flg"
  Cast115/1 -> Bus/298  "VINP_LaneMrkWidL_m"
  Cast116/1 -> Bus/299  "VINP_LaneMrkWidLValid_flg"
  Cast117/1 -> Bus/300  "VINP_LaneMrkWidR_m"
  Cast118/1 -> Bus/301  "VINP_LaneMrkWidRValid_flg"
  Cast119/1 -> Bus/302  "VINP_ViewRangeStartL_m"
  Cast120/1 -> Bus/303  "VINP_ViewRangeStartLValid_flg"
  Cast121/1 -> Bus/304  "VINP_ViewRangeStartR_m"
  Cast122/1 -> Bus/305  "VINP_ViewRangeStartRValid_flg"
  Cast123/1 -> Bus/310  "VINP_TLCL_s"
  Cast124/1 -> Bus/311  "VINP_TLCLValid_flg"
  Cast125/1 -> Bus/312  "VINP_TLCR_s"
  Cast126/1 -> Bus/313  "VINP_TLCRValid_flg"
  Cast127/1 -> Bus/314  "VINP_LaneCrosL_flg"
  Cast128/1 -> Bus/315  "VINP_LaneCrosLValid_flg"
  Cast129/1 -> Bus/316  "VINP_LaneCrosR_flg"
  Cast130/1 -> Bus/317  "VINP_LaneCrosRValid_flg"
  [METER_signal_bus] -> Terminator5/1
  METER_signal_bus/1 -> [METER_signal_bus]
  [AC_signal_bus] -> Terminator9/1
  AC_signal_bus/1 -> [AC_signal_bus]
  [EPB_signal_bus] -> Terminator10/1
  EPB_signal_bus/1 -> [EPB_signal_bus]
  [VCU_signal_bus] -> Terminator11/1
  VCU_signal_bus/1 -> [VCU_signal_bus]
  [HMI_signal_bus] -> Terminator12/1
  HMI_signal_bus/1 -> [HMI_signal_bus]
  Cast43/1 -> Bus/12  "VINP_AccelPedalPosn_pct"
  Cast45/1 -> Bus/13  "VINP_AccelPedalPosnValid_flg"
  Cast79/1 -> Bus/80  "VINP_ABSActive_flg"
  Cast80/1 -> Bus/82  "VINP_ABSFault_flg"
  Cast81/1 -> Bus/100  "VINP_BrkLampOn_flg"
  Cast82/1 -> Bus/101  "VINP_BrkLampOnValid_flg"
  Cast83/1 -> Bus/84  "VINP_EBDFault_flg"
  Cast84/1 -> Bus/86  "VINP_ESCActive_flg"
  Cast85/1 -> Bus/88  "VINP_ESCFault_flg"
  Cast86/1 -> Bus/90  "VINP_TCSActive_flg"
  Cast87/1 -> Bus/92  "VINP_TCSEnabled_flg"
  Cast88/1 -> Bus/94  "VINP_TCSFault_flg"
  Cast89/1 -> Bus/102  "VINP_VehSpd_kph"
  Cast90/1 -> Bus/103  "VINP_VehSpdValid_flg"
  Cast91/1 -> Bus/127  "VINP_SteerAngleValid_flg"
  Cast92/1 -> Bus/105  "VINP_YawRateValid_flg"
  Cast93/1 -> Bus/58  "VINP_KeySwitchStatus_enum"
  Cast94/1 -> Bus/60  "VINP_TurnSignalSwL_flg"
  Cast95/1 -> Bus/62  "VINP_TurnSignalSwR_flg"
  Cast181/1 -> Bus/40  "VINP_ShifterPosn_enum"
  Cast183/1 -> Bus/41  "VINP_ShifterPosnValid_flg"
  Cast186/1 -> Bus/134  "VINP_EPSElectPWR_A"
  [ECM_signal_bus] -> Bus Selector204/1
  Bus Selector204/1 -> Cast43/1  "<VINP_AccelPedalPosn_pct_InHAL>"
  Bus Selector204/2 -> Cast45/1  "<VINP_AccelPedalPosnValid_flg_InHAL>"
  [ABS_signal_bus] -> Bus Selector209/1
  Bus Selector209/1 -> Cast79/1  "<VINP_ABSActive_flg_InHAL>"
  Bus Selector7/6 -> Cast92/1  "<VINP_YawRateValid_flg_InHAL>"
  [BCM_signal_bus] -> Bus Selector226/1
  Bus Selector226/1 -> Cast93/1  "<VINP_KeySwitchStatus_enum_InHAL>"
  Bus Selector226/3 -> Cast94/1  "<VINP_TurnSignalSwL_flg_InHAL>"
  Bus Selector226/5 -> Cast95/1  "<VINP_TurnSignalSwR_flg_InHAL>"
  [TCM_signal_bus] -> Bus Selector230/1
  Bus Selector230/1 -> Cast181/1  "<VINP_ShifterPosn_enum_InHAL>"
  Bus Selector230/2 -> Cast183/1  "<VINP_ShifterPosnValid_flg_InHAL>"
  [EPS_signal_bus] -> Bus Selector232/1
  Bus Selector226/2 -> Cast192/1  "<VINP_KeySwitchStatusValid_flg_InHAL>"
  Cast192/1 -> Bus/59  "VINP_KeySwitchStatusValid_flg"
  Bus Selector226/4 -> Cast211/1  "<VINP_TurnSignalSwLValid_flg_InHAL>"
  Cast211/1 -> Bus/61  "VINP_TurnSignalSwLValid_flg"
  Bus Selector226/6 -> Cast212/1  "<VINP_TurnSignalSwRValid_flg_InHAL>"
  Cast212/1 -> Bus/63  "VINP_TurnSignalSwRValid_flg"
  Bus Selector209/2 -> Cast214/1  "<VINP_ABSActiveValid_flg_InHAL>"
  Bus Selector209/3 -> Cast80/1  "<VINP_ABSFault_flg_InHAL>"
  Bus Selector209/4 -> Cast215/1  "<VINP_ABSFaultValid_flg_InHAL>"
  Bus Selector209/5 -> Cast83/1  "<VINP_EBDFault_flg_InHAL>"
  Bus Selector209/6 -> Cast216/1  "<VINP_EBDFaultValid_flg_InHAL>"
  Bus Selector209/7 -> Cast84/1  "<VINP_ESCActive_flg_InHAL>"
  Bus Selector209/8 -> Cast217/1  "<VINP_ESCActiveValid_flg_InHAL>"
  Bus Selector209/9 -> Cast85/1  "<VINP_ESCFault_flg_InHAL>"
  Bus Selector209/10 -> Cast218/1  "<VINP_ESCFaultValid_flg_InHAL>"
  Bus Selector209/11 -> Cast86/1  "<VINP_TCSActive_flg_InHAL>"
  Bus Selector209/12 -> Cast219/1  "<VINP_TCSActiveValid_flg_InHAL>"
  Bus Selector209/13 -> Cast87/1  "<VINP_TCSEnabled_flg_InHAL>"
  Bus Selector209/14 -> Cast220/1  "<VINP_TCSEnabledValid_flg_InHAL>"
  Bus Selector209/15 -> Cast88/1  "<VINP_TCSFault_flg_InHAL>"
  [ABS_signal_bus] -> Bus Selector7/1
  Bus Selector7/1 -> Cast81/1  "<VINP_BrkLampOn_flg_InHAL>"
  Bus Selector7/2 -> Cast82/1  "<VINP_BrkLampOnValid_flg_InHAL>"
  Bus Selector7/3 -> Cast89/1  "<VINP_VehSpd_kph_InHAL>"
  Bus Selector7/4 -> Cast90/1  "<VINP_VehSpdValid_flg_InHAL>"
  Bus Selector41/1 -> Cast222/1  "<VINP_SteerAngle_deg_InHAL>"
  Bus Selector41/2 -> Cast91/1  "<VINP_SteerAngleValid_flg_InHAL>"
  Bus Selector7/5 -> Cast223/1  "<VINP_YawRate_degps_InHAL>"
  Cast214/1 -> Bus/81  "VINP_ABSActiveValid_flg"
  Cast215/1 -> Bus/83  "VINP_ABSFaultValid_flg"
  Cast216/1 -> Bus/85  "VINP_EBDFaultValid_flg"
  Cast217/1 -> Bus/87  "VINP_ESCActiveValid_flg"
  Cast218/1 -> Bus/89  "VINP_ESCFaultValid_flg"
  Cast219/1 -> Bus/91  "VINP_TCSActiveValid_flg"
  Cast220/1 -> Bus/93  "VINP_TCSEnabledValid_flg"
  Bus Selector209/16 -> Cast221/1  "<VINP_TCSFaultValid_flg_InHAL>"
  Cast221/1 -> Bus/95  "VINP_TCSFaultValid_flg"
  Cast222/1 -> Bus/126  "VINP_SteerAngle_deg"
  Cast223/1 -> Bus/104  "VINP_YawRate_degps"
  Bus Selector232/5 -> Cast186/1  "<VINP_EPSElectPWR_A_InHAL>"
  Bus Selector232/6 -> Cast226/1  "<VINP_EPSElectPWRValid_flg_InHAL>"
  Cast226/1 -> Bus/135  "VINP_EPSElectPWRValid_flg"
  Bus Selector204/16 -> Cast140/1  "<VINP_EngTqMinValid_flg_InHAL>"
  Bus Selector204/3 -> Cast131/1  "<VINP_EffAccelPedalPosn_pct_InHAL>"
  Cast131/1 -> Bus/14  "VINP_EffAccelPedalPosn_pct"
  Bus Selector204/4 -> Cast132/1  "<VINP_EffAccelPedalPosnValid_flg_InHAL>"
  Cast132/1 -> Bus/15  "VINP_EffAccelPedalPosnValid_flg"
  Bus Selector204/5 -> Cast133/1  "<VINP_EngSpd_erpm_InHAL>"
  Cast133/1 -> Bus/16  "VINP_EngSpd_erpm"
  Bus Selector204/6 -> Cast134/1  "<VINP_EngSpdValid_flg_InHAL>"
  Cast134/1 -> Bus/17  "VINP_EngSpdValid_flg"
  Bus Selector204/7 -> Cast135/1  "<VINP_EngTqFriction_Nm_InHAL>"
  Cast135/1 -> Bus/18  "VINP_EngTqFriction_Nm"
  Bus Selector204/8 -> Cast136/1  "<VINP_EngTqFrictionValid_flg_InHAL>"
  Cast136/1 -> Bus/19  "VINP_EngTqFrictionValid_flg"
  Bus Selector204/13 -> Cast137/1  "<VINP_EngTqMax_Nm_InHAL>"
  Cast137/1 -> Bus/24  "VINP_EngTqMax_Nm"
  Bus Selector204/14 -> Cast138/1  "<VINP_EngTqMaxValid_flg_InHAL>"
  Cast138/1 -> Bus/25  "VINP_EngTqMaxValid_flg"
  Bus Selector204/15 -> Cast139/1  "<VINP_EngTqMin_Nm_InHAL>"
  Cast139/1 -> Bus/26  "VINP_EngTqMin_Nm"
  Cast140/1 -> Bus/27  "VINP_EngTqMinValid_flg"
  Bus Selector232/3 -> Cast143/1  "<VINP_SteerTorque_Nm_InHAL>"
  Bus Selector232/4 -> Cast144/1  "<VINP_SteerTorqueValid_flg_InHAL>"
  Cast143/1 -> Bus/132  "VINP_SteerTorque_Nm"
  Cast144/1 -> Bus/133  "VINP_SteerTorqueValid_flg"
  Cast145/1 -> Bus/107  "VINP_LatAccelValid_flg"
  Cast146/1 -> Bus/106  "VINP_LatAccel_G"
  Cast147/1 -> Bus/109  "VINP_LongAccelValid_flg"
  Cast148/1 -> Bus/108  "VINP_LongAccel_G"
  Cast149/1 -> Bus/111  "VINP_MCPressureValid_flg"
  Cast150/1 -> Bus/110  "VINP_MCPressure_bar"
  Bus Selector7/7 -> Cast146/1  "<VINP_LatAccel_G_InHAL>"
  Bus Selector7/8 -> Cast145/1  "<VINP_LatAccelValid_flg_InHAL>"
  Bus Selector7/9 -> Cast148/1  "<VINP_LongAccel_G_InHAL>"
  Bus Selector7/10 -> Cast147/1  "<VINP_LongAccelValid_flg_InHAL>"
  Bus Selector7/11 -> Cast150/1  "<VINP_MCPressure_bar_InHAL>"
  Bus Selector7/12 -> Cast149/1  "<VINP_MCPressureValid_flg_InHAL>"
  Cast187/1 -> Bus/20  "VINP_EngTqIndicated_Nm"
  Cast188/1 -> Bus/21  "VINP_EngTqIndicatedValid_flg"
  Bus Selector204/9 -> Cast187/1  "<VINP_EngTqIndicated_Nm_InHAL>"
  Bus Selector204/10 -> Cast188/1  "<VINP_EngTqIndicatedValid_flg_InHAL>"
  Cast189/1 -> Bus/22  "VINP_EngTqIndicatedCOR_Nm"
  Cast190/1 -> Bus/23  "VINP_EngTqIndicatedCORValid_flg"
  Bus Selector204/11 -> Cast189/1  "<VINP_EngTqIndicatedCOR_Nm_InHAL>"
  Bus Selector204/12 -> Cast190/1  "<VINP_EngTqIndicatedCORValid_flg_InHAL>"
  Cast191/1 -> Bus/28  "VINP_EngTqUnCORFault_flg"
  Cast193/1 -> Bus/29  "VINP_EngTqUnCORFaultValid_flg"
  Bus Selector204/17 -> Cast191/1  "<VINP_EngTqUnCORFault_flg_InHAL>"
  Bus Selector204/18 -> Cast193/1  "<VINP_EngTqUnCORFaultValid_flg_InHAL>"
  Cast194/1 -> Bus/30  "VINP_EMSHybridOpState_enum"
  Cast195/1 -> Bus/31  "VINP_EMSHybridOpStateValid_flg"
  Bus Selector204/19 -> Cast194/1  "<VINP_EMSHybridOpState_enum_InHAL>"
  Bus Selector204/20 -> Cast195/1  "<VINP_EMSHybridOpStateValid_flg_InHAL>"
  Cast196/1 -> Bus/32  "VINP_EMSOpState_enum"
  Cast197/1 -> Bus/33  "VINP_EMSOpStateValid_flg"
  Bus Selector204/21 -> Cast196/1  "<VINP_EMSOpState_enum_InHAL>"
  Bus Selector204/22 -> Cast197/1  "<VINP_EMSOpStateValid_flg_InHAL>"
  Cast198/1 -> Bus/34  "VINP_EngHoodOpen_flg"
  Cast199/1 -> Bus/35  "VINP_EngHoodOpenValid_flg"
  Bus Selector204/23 -> Cast198/1  "<VINP_EngHoodOpen_flg_InHAL>"
  Bus Selector204/24 -> Cast199/1  "<VINP_EngHoodOpenValid_flg_InHAL>"
  Cast202/1 -> Bus/42  "VINP_NowGearPosn_enum"
  Cast203/1 -> Bus/43  "VINP_NowGearPosnValid_flg"
  Bus Selector230/3 -> Cast202/1  "<VINP_NowGearPosn_enum_InHAL>"
  Bus Selector230/4 -> Cast203/1  "<VINP_NowGearPosnValid_flg_InHAL>"
  Cast204/1 -> Bus/44  "VINP_TMTurbineSpd_rpm"
  Cast205/1 -> Bus/45  "VINP_TMTurbineSpdValid_flg"
  Bus Selector230/5 -> Cast204/1  "<VINP_TMTurbineSpd_rpm_InHAL>"
  Bus Selector230/6 -> Cast205/1  "<VINP_TMTurbineSpdValid_flg_InHAL>"
  Cast206/1 -> Bus/46  "VINP_TCMFaultStatus_enum"
  Cast207/1 -> Bus/47  "VINP_TCMFaultStatusValid_flg"
  Bus Selector230/7 -> Cast206/1  "<VINP_TCMFaultStatus_enum_InHAL>"
  Bus Selector230/8 -> Cast207/1  "<VINP_TCMFaultStatusValid_flg_InHAL>"
  Cast208/1 -> Bus/56  "VINP_DriverMode_enum"
  Cast209/1 -> Bus/57  "VINP_DriverModeValid_flg"
  Bus Selector230/17 -> Cast208/1  "<VINP_DriverMode_enum_InHAL>"
  Bus Selector230/18 -> Cast209/1  "<VINP_DriverModeValid_flg_InHAL>"
  Cast210/1 -> Bus/64  "VINP_HazardLightSw_flg"
  Cast213/1 -> Bus/65  "VINP_HazardLightSwValid_flg"
  Bus Selector226/7 -> Cast210/1  "<VINP_HazardLightSw_flg_InHAL>"
  Bus Selector226/8 -> Cast213/1  "<VINP_HazardLightSwValid_flg_InHAL>"
  Cast227/1 -> Bus/66  "VINP_DriverDoorOpen_flg"
  Cast228/1 -> Bus/67  "VINP_DriverDoorOpenValid_flg"
  Bus Selector226/9 -> Cast227/1  "<VINP_DriverDoorOpen_flg_InHAL>"
  Bus Selector226/10 -> Cast228/1  "<VINP_DriverDoorOpenValid_flg_InHAL>"
  Cast229/1 -> Bus/68  "VINP_PassengerDoorOpen_flg"